simulink model "ClimaTiMgr"  (R2022b)
settings: Solver=FixedStepDiscrete  StartTime=0.0  StopTime=10.0  FixedStep=0.1  RelTol=1e-3
settings Model: Creator=blue.ge  LastModifiedBy=blue.ge
settings DataIOCC: SaveFormat=StructureWithTime
settings OptimizationCC: DefaultParameterBehavior=Inlined  EnhancedBackFolding=on  PassReuseOutputArgsAs=Individual arguments  ZeroExternalMemoryAtStartup=off  ZeroInternalMemoryAtStartup=off  OptimizeBlockOrder=speed  DifferentSizesBufferReuse=on  OptimizationCustomize=off  EfficientTunableParamExpr=on
settings DebuggingCC: ParameterTunabilityLossMsg=error
settings ModelReferenceCC: ModelReferenceNumInstancesAllowed=Single
settings SFSimCC: MATLABDynamicMemAlloc=off
settings RTWCC: Description=AUTOSAR  SystemTargetFile=autosar.tlc  GenCodeOnly=on  TemplateMakefile=ert_default_tmf
settings CodeAppCC: ForceParamTrailComments=on  IgnoreCustomStorageClasses=off  OperatorAnnotations=on  SimulinkDataObjDesc=on  SFDataObjDesc=on  InsertBlockDesc=on
settings STFCustomTargetCC: TargetLangStandard=C99 (ISO)  MultiwordLength=256  ParMdlRefBuildCompliant=on  CompOptLevelCompliant=on  IncludeMdlTerminateFcn=off  CombineOutputUpdateFcns=on  SuppressErrorStatus=on  ERTCustomFileBanners=on  SupportNonFinite=off  SupportComplex=off  SupportContinuousTime=off  SupportNonInlinedSFcns=off  RemoveResetFunc=on  MATLABClassNameForMDSCustomization=Simulink.SoftwareTarget.ERTCustomization  AutosarCompliant=on  UseToolchainInfoCompliant=on  LookupTableObjectStructAxisOrder=2,1,3,4,...  LUTObjectStructOrderEvenSpacing=Size,Table,Breakpoints  SystemTargetFile=autosar.tlc
settings ERTTargetCC: TargetLangStandard=C99 (ISO)  ConcurrentExecutionCompliant=off  IncludeMdlTerminateFcn=off  SuppressErrorStatus=on  SupportNonFinite=off  SupportComplex=off  ModelStepFunctionPrototypeControlCompliant=off  CPPClassGenCompliant=off  AutosarCompliant=on  LookupTableObjectStructAxisOrder=2,1,3,4,...  LUTObjectStructOrderEvenSpacing=Size,Table,Breakpoints  InlinedParameterPlacement=NonHierarchical  ERTCustomFileTemplate=example_file_process.tlc

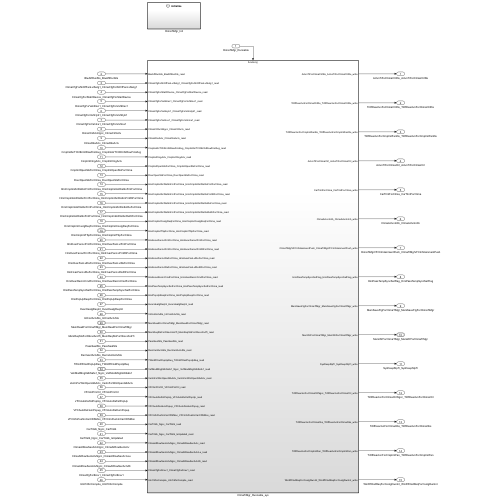
[thumbnail: Simulink's stored preview of the top-level diagram]
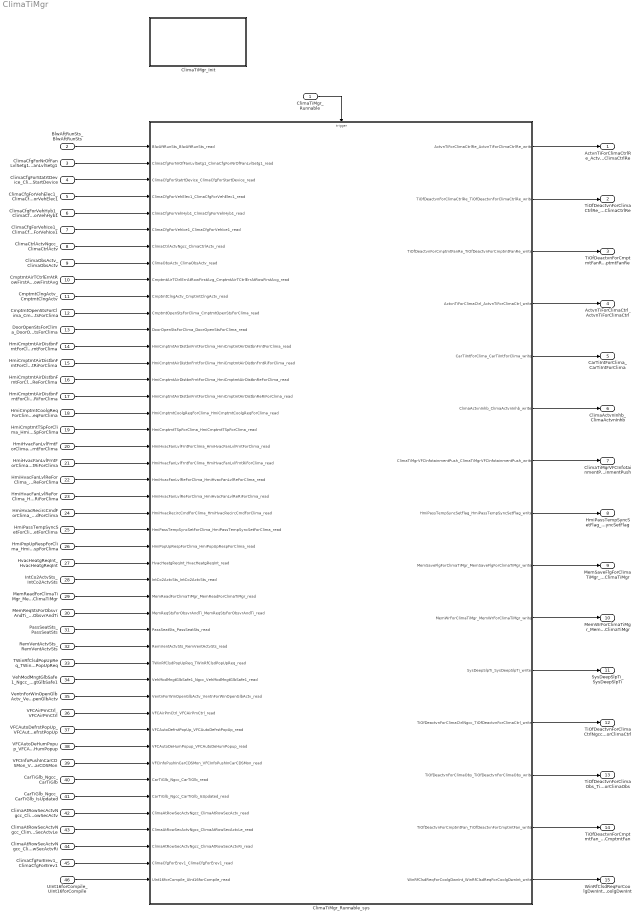
== system ClimaTiMgr ==
  ClimaTiMgr_Runnable : Inport  SampleTime=0.1  OutputFunctionCall=on
  BlwAftRunSts_BlwAftRunSts : Inport  Port=2  OutDataTypeStr=Boolean  PortDimensions=1
  ClimaCfgForNrOfFanLvlSetg1_ClimaCfgForNrOfFanLvlSetg1 : Inport  Port=3  OutDataTypeStr=Enum: ClimaCfgForNrOfFanLvlSetg1  PortDimensions=1
  ClimaCfgForStatrtDevice_ClimaCfgForStartDevice : Inport  Port=4  OutDataTypeStr=Enum: StartDevice  PortDimensions=1
  ClimaCfgForVehElec1_ClimaCfgForVehElec1 : Inport  Port=5  OutDataTypeStr=Boolean  PortDimensions=1
  ClimaCfgForVehHyb1_ClimaCfgForVehHyb1 : Inport  Port=6  OutDataTypeStr=Boolean  PortDimensions=1
  ClimaCfgForVehIce1_ClimaCfgForVehIce1 : Inport  Port=7  OutDataTypeStr=Boolean  PortDimensions=1
  ClimaCtrlActvNgcc_ClimaCtrlActv : Inport  Port=8  OutDataTypeStr=Boolean  PortDimensions=1
  ClimaObsActv_ClimaObsActv : Inport  Port=9  OutDataTypeStr=Boolean  PortDimensions=1
  CmptmtAirTCtrlErrAtRowFirstAvg_CmptmtAirTCtrlErrAtRowFirstAvg : Inport  Port=10  OutDataTypeStr=T30  PortDimensions=1
  CmptmtClngActv_CmptmtClngActv : Inport  Port=11  OutDataTypeStr=Enum: CmptmtClngActv  PortDimensions=1
  CmptmtOpenStsForClima_CmptmtOpenStsForClima : Inport  Port=12  OutDataTypeStr=Boolean  PortDimensions=1
  DoorOpenStsForClima_DoorOpenStsForClima : Inport  Port=13  OutDataTypeStr=Boolean  PortDimensions=1
  HmiCmptmtAirDistbnFrntForClima_HmiCmptmtAirDistbnFrntForClima : Inport  Port=14  OutDataTypeStr=Enum: HmiCmptmtAirDistbnFrnt  PortDimensions=1
  HmiCmptmtAirDistbnFrntForClima_HmiCmptmtAirDistbnFrntRiForClima : Inport  Port=15  OutDataTypeStr=Enum: HmiCmptmtAirDistbnFrnt  PortDimensions=1
  HmiCmptmtAirDistbnFrntForClima_HmiCmptmtAirDistbnReForClima : Inport  Port=16  OutDataTypeStr=Enum: HmiCmptmtAirDistbnFrnt  PortDimensions=1
  HmiCmptmtAirDistbnFrntForClima_HmiCmptmtAirDistbnReRiForClima : Inport  Port=17  OutDataTypeStr=Enum: HmiCmptmtAirDistbnFrnt  PortDimensions=1
  HmiCmptmtCoolgReqForClima_HmiCmptmtCoolgReqForClima : Inport  Port=18  OutDataTypeStr=Enum: HmiCmptmtCoolgReq  PortDimensions=1
  HmiCmptmtTSpForClima_HmiCmptmtTSpForClima : Inport  Port=19  OutDataTypeStr=Bus: HmiCmptmtTSp  PortDimensions=1  BusOutputAsStruct=on
  HmiHvacFanLvlFrntForClima_HmiHvacFanLvlFrntForClima : Inport  Port=20  OutDataTypeStr=Enum: HmiHvacFanLvl  PortDimensions=1
  HmiHvacFanLvlFrntForClima_HmiHvacFanLvlFrntRiForClima : Inport  Port=21  OutDataTypeStr=Enum: HmiHvacFanLvl  PortDimensions=1
  HmiHvacFanLvlReForClima_HmiHvacFanLvlReForClima : Inport  Port=22  OutDataTypeStr=Enum: HmiHvacFanLvl  PortDimensions=1
  HmiHvacFanLvlReForClima_HmiHvacFanLvlReRiForClima : Inport  Port=23  OutDataTypeStr=Enum: HmiHvacFanLvl  PortDimensions=1
  HmiHvacRecircCmdForClima_HmiHvacRecircCmdForClima : Inport  Port=24  OutDataTypeStr=Enum: HmiHvacRecircCmd  PortDimensions=1
  HmiPassTempSyncSetForClima_HmiPassTempSyncSetForClima : Inport  Port=25  OutDataTypeStr=Enum: OnOff1  PortDimensions=1
  HmiPopUpRespForClima_HmiPopUpRespForClima : Inport  Port=26  OutDataTypeStr=Enum: BookChargeSetResponse  PortDimensions=1
  HvacHeatgReqInt_HvacHeatgReqInt : Inport  Port=27  OutDataTypeStr=Enum: OnOff1  PortDimensions=1
  IntCo2ActvSts_IntCo2ActvSts : Inport  Port=28  OutDataTypeStr=Enum: IntCo2Sts  PortDimensions=1
  MemReadForClimaTiMgr_MemReadForClimaTiMgr : Inport  Port=29  OutDataTypeStr=Bus: MemRecForClimaTiMgr  PortDimensions=1  BusOutputAsStruct=on
  MemReqStsForObsvrAndTi_MemReqStsForObsvrAndTi : Inport  Port=30  OutDataTypeStr=Enum: MemReqSts  PortDimensions=1
  PassSeatSts_PassSeatSts : Inport  Port=31  OutDataTypeStr=Enum: PassSeatSts  PortDimensions=1
  RemVentActvSts_RemVentActvSts : Inport  Port=32  OutDataTypeStr=Enum: OnOff1  PortDimensions=1
  TWinRfClsdPopUpReq_TWinRfClsdPopUpReq : Inport  Port=33  OutDataTypeStr=Enum: OnOff1  PortDimensions=1
  VehModMngtGlbSafe1_Ngcc_VehModMngtGlbSafe1 : Inport  Port=34  OutDataTypeStr=Bus: VehModMngtGlbSafe1  PortDimensions=1  BusOutputAsStruct=on
  VentnForWinOpenGlbActv_VentnForWinOpenGlbActv : Inport  Port=35  OutDataTypeStr=Boolean  PortDimensions=1
  VFCAirPmCtrl_VFCAirPmCtrl : Inport  Port=36  OutDataTypeStr=Enum: OnOff1  PortDimensions=1
  VFCAutoDefrstPopUp_VFCAutoDefrstPopUp : Inport  Port=37  OutDataTypeStr=Boolean  PortDimensions=1
  VFCAutoDeHumPopup_VFCAutoDeHumPopup : Inport  Port=38  OutDataTypeStr=Boolean  PortDimensions=1
  VFCInfoPushInCarCDSMon_VFCInfoPushInCarCDSMon : Inport  Port=39  OutDataTypeStr=Boolean  PortDimensions=1
  CarTiGlb_Ngcc_CarTiGlb : Inport  Port=40  OutDataTypeStr=CarTiGlb  PortDimensions=1
  CarTiGlb_Ngcc_CarTiGlb_IsUpdated : Inport  Port=41  OutDataTypeStr=boolean  PortDimensions=1
  ClimaAtRowSecActvNgcc_ClimaAtRowSecActv : Inport  Port=42  OutDataTypeStr=Boolean  PortDimensions=1
  ClimaAtRowSecActvNgcc_ClimaAtRowSecActvLe : Inport  Port=43  OutDataTypeStr=Boolean  PortDimensions=1
  ClimaAtRowSecActvNgcc_ClimaAtRowSecActvRi : Inport  Port=44  OutDataTypeStr=Boolean  PortDimensions=1
  ClimaCfgForErev1_ClimaCfgForErev1 : Inport  Port=45  OutDataTypeStr=Boolean  PortDimensions=1
  UInt16forCompile_UInt16forCompile : Inport  Port=46  OutDataTypeStr=UInt16  PortDimensions=1
  ClimaTiMgr_Init : Subsystem
  ClimaTiMgr_Runnable_sys : Subsystem
  ActvnTiForClimaCtrlRe_ActvnTiForClimaCtrlRe : Outport  OutDataTypeStr=Ti33  PortDimensions=1
  TiOfDeactvnForClimaCtrlRe_TiOfDeactvnForClimaCtrlRe : Outport  Port=2  OutDataTypeStr=Ti33  PortDimensions=1
  TiOfDeactvnForCmptmtFanRe_TiOfDeactvnForCmptmtFanRe : Outport  Port=3  OutDataTypeStr=Ti33  PortDimensions=1
  ActvnTiForClimaCtrl_ActvnTiForClimaCtrl : Outport  Port=4  OutDataTypeStr=Ti33  PortDimensions=1
  CarTiIntForClima_CarTiIntForClima : Outport  Port=5  OutDataTypeStr=CarTiGlb  PortDimensions=1
  ClimaActvnInhb_ClimaActvnInhb : Outport  Port=6  OutDataTypeStr=Enum: OnOff1  PortDimensions=1
  ClimaTiMgrVFCInfotainmentPush_ClimaTiMgrVFCInfotainmentPush : Outport  Port=7  OutDataTypeStr=Enum: OnOff1  PortDimensions=1
  HmiPassTempSyncSetFlag_HmiPassTempSyncSetFlag : Outport  Port=8  OutDataTypeStr=Boolean  PortDimensions=1
  MemSaveFlgForClimaTiMgr_MemSaveFlgForClimaTiMgr : Outport  Port=9  OutDataTypeStr=Boolean  PortDimensions=1
  MemWrForClimaTiMgr_MemWrForClimaTiMgr : Outport  Port=10  OutDataTypeStr=Bus: MemRecForClimaTiMgr  PortDimensions=1  BusOutputAsStruct=on
  SysDeepSlpTi_SysDeepSlpTi : Outport  Port=11  OutDataTypeStr=CarTiGlb  PortDimensions=1
  TiOfDeactvnForClimaCtrlNgcc_TiOfDeactvnForClimaCtrl : Outport  Port=12  OutDataTypeStr=Ti33  PortDimensions=1
  TiOfDeactvnForClimaObs_TiOfDeactvnForClimaObs : Outport  Port=13  OutDataTypeStr=Ti33  PortDimensions=1
  TiOfDeactvnForCmptmtFan_TiOfDeactvnForCmptmtFan : Outport  Port=14  OutDataTypeStr=Ti33  PortDimensions=1
  WinRfClsdReqForCoolgDwnInt_WinRfClsdReqForCoolgDwnInt : Outport  Port=15  OutDataTypeStr=Enum: Warn2  PortDimensions=1
  wires:
  BlwAftRunSts_BlwAftRunSts/1 -> ClimaTiMgr_Runnable_sys/1(BlwAftRunSts_BlwAftRunSts_read)
  ClimaCfgForNrOfFanLvlSetg1_ClimaCfgForNrOfFanLvlSetg1/1 -> ClimaTiMgr_Runnable_sys/2(ClimaCfgForNrOfFanLvlSetg1_ClimaCfgForNrOfFanLvlSetg1_read)
  ClimaCfgForStatrtDevice_ClimaCfgForStartDevice/1 -> ClimaTiMgr_Runnable_sys/3(ClimaCfgForStatrtDevice_ClimaCfgForStartDevice_read)
  ClimaCfgForVehElec1_ClimaCfgForVehElec1/1 -> ClimaTiMgr_Runnable_sys/4(ClimaCfgForVehElec1_ClimaCfgForVehElec1_read)
  ClimaCfgForVehHyb1_ClimaCfgForVehHyb1/1 -> ClimaTiMgr_Runnable_sys/5(ClimaCfgForVehHyb1_ClimaCfgForVehHyb1_read)
  ClimaCfgForVehIce1_ClimaCfgForVehIce1/1 -> ClimaTiMgr_Runnable_sys/6(ClimaCfgForVehIce1_ClimaCfgForVehIce1_read)
  ClimaCtrlActvNgcc_ClimaCtrlActv/1 -> ClimaTiMgr_Runnable_sys/7(ClimaCtrlActvNgcc_ClimaCtrlActv_read)
  ClimaObsActv_ClimaObsActv/1 -> ClimaTiMgr_Runnable_sys/8(ClimaObsActv_ClimaObsActv_read)
  CmptmtAirTCtrlErrAtRowFirstAvg_CmptmtAirTCtrlErrAtRowFirstAvg/1 -> ClimaTiMgr_Runnable_sys/9(CmptmtAirTCtrlErrAtRowFirstAvg_CmptmtAirTCtrlErrAtRowFirstAvg_read)
  CmptmtClngActv_CmptmtClngActv/1 -> ClimaTiMgr_Runnable_sys/10(CmptmtClngActv_CmptmtClngActv_read)
  CmptmtOpenStsForClima_CmptmtOpenStsForClima/1 -> ClimaTiMgr_Runnable_sys/11(CmptmtOpenStsForClima_CmptmtOpenStsForClima_read)
  DoorOpenStsForClima_DoorOpenStsForClima/1 -> ClimaTiMgr_Runnable_sys/12(DoorOpenStsForClima_DoorOpenStsForClima_read)
  HmiCmptmtAirDistbnFrntForClima_HmiCmptmtAirDistbnFrntForClima/1 -> ClimaTiMgr_Runnable_sys/13(HmiCmptmtAirDistbnFrntForClima_HmiCmptmtAirDistbnFrntForClima_read)
  HmiCmptmtAirDistbnFrntForClima_HmiCmptmtAirDistbnFrntRiForClima/1 -> ClimaTiMgr_Runnable_sys/14(HmiCmptmtAirDistbnFrntForClima_HmiCmptmtAirDistbnFrntRiForClima_read)
  HmiCmptmtAirDistbnFrntForClima_HmiCmptmtAirDistbnReForClima/1 -> ClimaTiMgr_Runnable_sys/15(HmiCmptmtAirDistbnFrntForClima_HmiCmptmtAirDistbnReForClima_read)
  HmiCmptmtAirDistbnFrntForClima_HmiCmptmtAirDistbnReRiForClima/1 -> ClimaTiMgr_Runnable_sys/16(HmiCmptmtAirDistbnFrntForClima_HmiCmptmtAirDistbnReRiForClima_read)
  HmiCmptmtCoolgReqForClima_HmiCmptmtCoolgReqForClima/1 -> ClimaTiMgr_Runnable_sys/17(HmiCmptmtCoolgReqForClima_HmiCmptmtCoolgReqForClima_read)
  HmiCmptmtTSpForClima_HmiCmptmtTSpForClima/1 -> ClimaTiMgr_Runnable_sys/18(HmiCmptmtTSpForClima_HmiCmptmtTSpForClima_read)
  HmiHvacFanLvlFrntForClima_HmiHvacFanLvlFrntForClima/1 -> ClimaTiMgr_Runnable_sys/19(HmiHvacFanLvlFrntForClima_HmiHvacFanLvlFrntForClima_read)
  HmiHvacFanLvlFrntForClima_HmiHvacFanLvlFrntRiForClima/1 -> ClimaTiMgr_Runnable_sys/20(HmiHvacFanLvlFrntForClima_HmiHvacFanLvlFrntRiForClima_read)
  HmiHvacFanLvlReForClima_HmiHvacFanLvlReForClima/1 -> ClimaTiMgr_Runnable_sys/21(HmiHvacFanLvlReForClima_HmiHvacFanLvlReForClima_read)
  HmiHvacFanLvlReForClima_HmiHvacFanLvlReRiForClima/1 -> ClimaTiMgr_Runnable_sys/22(HmiHvacFanLvlReForClima_HmiHvacFanLvlReRiForClima_read)
  HmiHvacRecircCmdForClima_HmiHvacRecircCmdForClima/1 -> ClimaTiMgr_Runnable_sys/23(HmiHvacRecircCmdForClima_HmiHvacRecircCmdForClima_read)
  HmiPassTempSyncSetForClima_HmiPassTempSyncSetForClima/1 -> ClimaTiMgr_Runnable_sys/24(HmiPassTempSyncSetForClima_HmiPassTempSyncSetForClima_read)
  HmiPopUpRespForClima_HmiPopUpRespForClima/1 -> ClimaTiMgr_Runnable_sys/25(HmiPopUpRespForClima_HmiPopUpRespForClima_read)
  HvacHeatgReqInt_HvacHeatgReqInt/1 -> ClimaTiMgr_Runnable_sys/26(HvacHeatgReqInt_HvacHeatgReqInt_read)
  IntCo2ActvSts_IntCo2ActvSts/1 -> ClimaTiMgr_Runnable_sys/27(IntCo2ActvSts_IntCo2ActvSts_read)
  MemReadForClimaTiMgr_MemReadForClimaTiMgr/1 -> ClimaTiMgr_Runnable_sys/28(MemReadForClimaTiMgr_MemReadForClimaTiMgr_read)
  MemReqStsForObsvrAndTi_MemReqStsForObsvrAndTi/1 -> ClimaTiMgr_Runnable_sys/29(MemReqStsForObsvrAndTi_MemReqStsForObsvrAndTi_read)
  PassSeatSts_PassSeatSts/1 -> ClimaTiMgr_Runnable_sys/30(PassSeatSts_PassSeatSts_read)
  RemVentActvSts_RemVentActvSts/1 -> ClimaTiMgr_Runnable_sys/31(RemVentActvSts_RemVentActvSts_read)
  TWinRfClsdPopUpReq_TWinRfClsdPopUpReq/1 -> ClimaTiMgr_Runnable_sys/32(TWinRfClsdPopUpReq_TWinRfClsdPopUpReq_read)
  VehModMngtGlbSafe1_Ngcc_VehModMngtGlbSafe1/1 -> ClimaTiMgr_Runnable_sys/33(VehModMngtGlbSafe1_Ngcc_VehModMngtGlbSafe1_read)
  VentnForWinOpenGlbActv_VentnForWinOpenGlbActv/1 -> ClimaTiMgr_Runnable_sys/34(VentnForWinOpenGlbActv_VentnForWinOpenGlbActv_read)
  VFCAirPmCtrl_VFCAirPmCtrl/1 -> ClimaTiMgr_Runnable_sys/35(VFCAirPmCtrl_VFCAirPmCtrl_read)
  VFCAutoDefrstPopUp_VFCAutoDefrstPopUp/1 -> ClimaTiMgr_Runnable_sys/36(VFCAutoDefrstPopUp_VFCAutoDefrstPopUp_read)
  VFCAutoDeHumPopup_VFCAutoDeHumPopup/1 -> ClimaTiMgr_Runnable_sys/37(VFCAutoDeHumPopup_VFCAutoDeHumPopup_read)
  VFCInfoPushInCarCDSMon_VFCInfoPushInCarCDSMon/1 -> ClimaTiMgr_Runnable_sys/38(VFCInfoPushInCarCDSMon_VFCInfoPushInCarCDSMon_read)
  CarTiGlb_Ngcc_CarTiGlb/1 -> ClimaTiMgr_Runnable_sys/39(CarTiGlb_Ngcc_CarTiGlb_read)
  CarTiGlb_Ngcc_CarTiGlb_IsUpdated/1 -> ClimaTiMgr_Runnable_sys/40(CarTiGlb_Ngcc_CarTiGlb_IsUpdated_read)
  ClimaAtRowSecActvNgcc_ClimaAtRowSecActv/1 -> ClimaTiMgr_Runnable_sys/41(ClimaAtRowSecActvNgcc_ClimaAtRowSecActv_read)
  ClimaAtRowSecActvNgcc_ClimaAtRowSecActvLe/1 -> ClimaTiMgr_Runnable_sys/42(ClimaAtRowSecActvNgcc_ClimaAtRowSecActvLe_read)
  ClimaAtRowSecActvNgcc_ClimaAtRowSecActvRi/1 -> ClimaTiMgr_Runnable_sys/43(ClimaAtRowSecActvNgcc_ClimaAtRowSecActvRi_read)
  ClimaCfgForErev1_ClimaCfgForErev1/1 -> ClimaTiMgr_Runnable_sys/44(ClimaCfgForErev1_ClimaCfgForErev1_read)
  UInt16forCompile_UInt16forCompile/1 -> ClimaTiMgr_Runnable_sys/45(UInt16forCompile_UInt16forCompile_read)
  ClimaTiMgr_Runnable_sys/1(ActvnTiForClimaCtrlRe_ActvnTiForClimaCtrlRe_write) -> ActvnTiForClimaCtrlRe_ActvnTiForClimaCtrlRe/1
  ClimaTiMgr_Runnable_sys/2(TiOfDeactvnForClimaCtrlRe_TiOfDeactvnForClimaCtrlRe_write) -> TiOfDeactvnForClimaCtrlRe_TiOfDeactvnForClimaCtrlRe/1
  ClimaTiMgr_Runnable_sys/3(TiOfDeactvnForCmptmtFanRe_TiOfDeactvnForCmptmtFanRe_write) -> TiOfDeactvnForCmptmtFanRe_TiOfDeactvnForCmptmtFanRe/1
  ClimaTiMgr_Runnable_sys/4(ActvnTiForClimaCtrl_ActvnTiForClimaCtrl_write) -> ActvnTiForClimaCtrl_ActvnTiForClimaCtrl/1
  ClimaTiMgr_Runnable_sys/5(CarTiIntForClima_CarTiIntForClima_write) -> CarTiIntForClima_CarTiIntForClima/1
  ClimaTiMgr_Runnable_sys/6(ClimaActvnInhb_ClimaActvnInhb_write) -> ClimaActvnInhb_ClimaActvnInhb/1
  ClimaTiMgr_Runnable_sys/7(ClimaTiMgrVFCInfotainmentPush_ClimaTiMgrVFCInfotainmentPush_write) -> ClimaTiMgrVFCInfotainmentPush_ClimaTiMgrVFCInfotainmentPush/1
  ClimaTiMgr_Runnable_sys/8(HmiPassTempSyncSetFlag_HmiPassTempSyncSetFlag_write) -> HmiPassTempSyncSetFlag_HmiPassTempSyncSetFlag/1
  ClimaTiMgr_Runnable_sys/9(MemSaveFlgForClimaTiMgr_MemSaveFlgForClimaTiMgr_write) -> MemSaveFlgForClimaTiMgr_MemSaveFlgForClimaTiMgr/1
  ClimaTiMgr_Runnable_sys/10(MemWrForClimaTiMgr_MemWrForClimaTiMgr_write) -> MemWrForClimaTiMgr_MemWrForClimaTiMgr/1
  ClimaTiMgr_Runnable_sys/11(SysDeepSlpTi_SysDeepSlpTi_write) -> SysDeepSlpTi_SysDeepSlpTi/1
  ClimaTiMgr_Runnable_sys/12(TiOfDeactvnForClimaCtrlNgcc_TiOfDeactvnForClimaCtrl_write) -> TiOfDeactvnForClimaCtrlNgcc_TiOfDeactvnForClimaCtrl/1
  ClimaTiMgr_Runnable_sys/13(TiOfDeactvnForClimaObs_TiOfDeactvnForClimaObs_write) -> TiOfDeactvnForClimaObs_TiOfDeactvnForClimaObs/1
  ClimaTiMgr_Runnable_sys/14(TiOfDeactvnForCmptmtFan_TiOfDeactvnForCmptmtFan_write) -> TiOfDeactvnForCmptmtFan_TiOfDeactvnForCmptmtFan/1
  ClimaTiMgr_Runnable_sys/15(WinRfClsdReqForCoolgDwnInt_WinRfClsdReqForCoolgDwnInt_write) -> WinRfClsdReqForCoolgDwnInt_WinRfClsdReqForCoolgDwnInt/1
  ClimaTiMgr_Runnable/1 -> ClimaTiMgr_Runnable_sys/Trigger

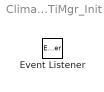
== system ClimaTiMgr/ClimaTiMgr_Init ==
  Event Listener : EventListener  EventName=reset

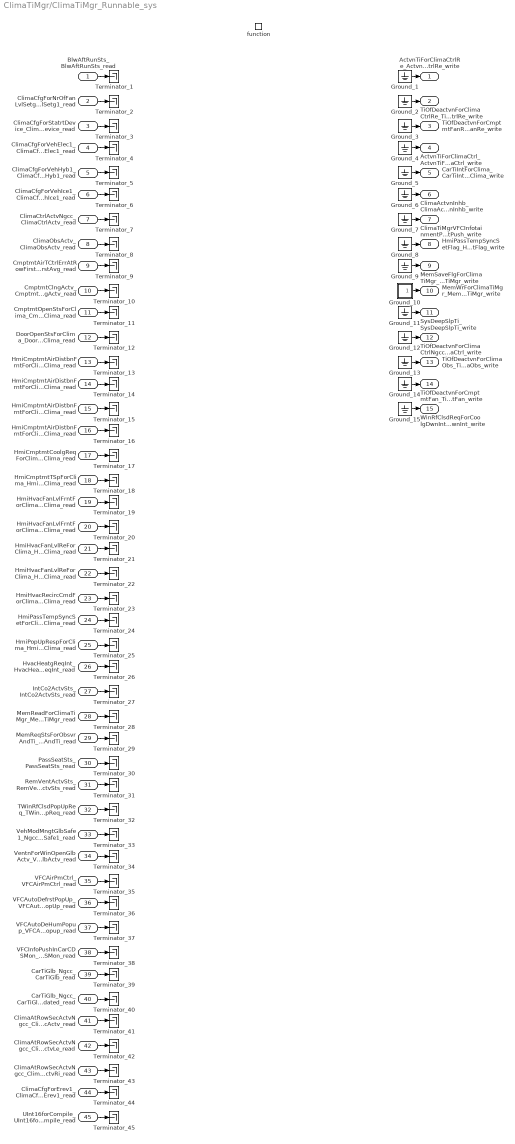
== system ClimaTiMgr/ClimaTiMgr_Runnable_sys ==
  BlwAftRunSts_BlwAftRunSts_read : Inport
  ClimaCfgForNrOfFanLvlSetg1_ClimaCfgForNrOfFanLvlSetg1_read : Inport  Port=2
  ClimaCfgForStatrtDevice_ClimaCfgForStartDevice_read : Inport  Port=3
  ClimaCfgForVehElec1_ClimaCfgForVehElec1_read : Inport  Port=4
  ClimaCfgForVehHyb1_ClimaCfgForVehHyb1_read : Inport  Port=5
  ClimaCfgForVehIce1_ClimaCfgForVehIce1_read : Inport  Port=6
  ClimaCtrlActvNgcc_ClimaCtrlActv_read : Inport  Port=7
  ClimaObsActv_ClimaObsActv_read : Inport  Port=8
  CmptmtAirTCtrlErrAtRowFirstAvg_CmptmtAirTCtrlErrAtRowFirstAvg_read : Inport  Port=9
  CmptmtClngActv_CmptmtClngActv_read : Inport  Port=10
  CmptmtOpenStsForClima_CmptmtOpenStsForClima_read : Inport  Port=11
  DoorOpenStsForClima_DoorOpenStsForClima_read : Inport  Port=12
  HmiCmptmtAirDistbnFrntForClima_HmiCmptmtAirDistbnFrntForClima_read : Inport  Port=13
  HmiCmptmtAirDistbnFrntForClima_HmiCmptmtAirDistbnFrntRiForClima_read : Inport  Port=14
  HmiCmptmtAirDistbnFrntForClima_HmiCmptmtAirDistbnReForClima_read : Inport  Port=15
  HmiCmptmtAirDistbnFrntForClima_HmiCmptmtAirDistbnReRiForClima_read : Inport  Port=16
  HmiCmptmtCoolgReqForClima_HmiCmptmtCoolgReqForClima_read : Inport  Port=17
  HmiCmptmtTSpForClima_HmiCmptmtTSpForClima_read : Inport  Port=18
  HmiHvacFanLvlFrntForClima_HmiHvacFanLvlFrntForClima_read : Inport  Port=19
  HmiHvacFanLvlFrntForClima_HmiHvacFanLvlFrntRiForClima_read : Inport  Port=20
  HmiHvacFanLvlReForClima_HmiHvacFanLvlReForClima_read : Inport  Port=21
  HmiHvacFanLvlReForClima_HmiHvacFanLvlReRiForClima_read : Inport  Port=22
  HmiHvacRecircCmdForClima_HmiHvacRecircCmdForClima_read : Inport  Port=23
  HmiPassTempSyncSetForClima_HmiPassTempSyncSetForClima_read : Inport  Port=24
  HmiPopUpRespForClima_HmiPopUpRespForClima_read : Inport  Port=25
  HvacHeatgReqInt_HvacHeatgReqInt_read : Inport  Port=26
  IntCo2ActvSts_IntCo2ActvSts_read : Inport  Port=27
  MemReadForClimaTiMgr_MemReadForClimaTiMgr_read : Inport  Port=28
  MemReqStsForObsvrAndTi_MemReqStsForObsvrAndTi_read : Inport  Port=29
  PassSeatSts_PassSeatSts_read : Inport  Port=30
  RemVentActvSts_RemVentActvSts_read : Inport  Port=31
  TWinRfClsdPopUpReq_TWinRfClsdPopUpReq_read : Inport  Port=32
  VehModMngtGlbSafe1_Ngcc_VehModMngtGlbSafe1_read : Inport  Port=33
  VentnForWinOpenGlbActv_VentnForWinOpenGlbActv_read : Inport  Port=34
  VFCAirPmCtrl_VFCAirPmCtrl_read : Inport  Port=35
  VFCAutoDefrstPopUp_VFCAutoDefrstPopUp_read : Inport  Port=36
  VFCAutoDeHumPopup_VFCAutoDeHumPopup_read : Inport  Port=37
  VFCInfoPushInCarCDSMon_VFCInfoPushInCarCDSMon_read : Inport  Port=38
  CarTiGlb_Ngcc_CarTiGlb_read : Inport  Port=39
  CarTiGlb_Ngcc_CarTiGlb_IsUpdated_read : Inport  Port=40
  ClimaAtRowSecActvNgcc_ClimaAtRowSecActv_read : Inport  Port=41
  ClimaAtRowSecActvNgcc_ClimaAtRowSecActvLe_read : Inport  Port=42
  ClimaAtRowSecActvNgcc_ClimaAtRowSecActvRi_read : Inport  Port=43
  ClimaCfgForErev1_ClimaCfgForErev1_read : Inport  Port=44
  UInt16forCompile_UInt16forCompile_read : Inport  Port=45
  function : TriggerPort  TriggerType=function-call  VariantControl=(inherit)  SampleTimeType=periodic  SampleTime=-1
  Ground_{1..9,11..15} (14 alike) : Ground
  Ground_10 : Subsystem
  Terminator_{1..45} (45 alike) : Terminator
  ActvnTiForClimaCtrlRe_ActvnTiForClimaCtrlRe_write : Outport
      settings: EnsureOutportIsVirtual=on
  TiOfDeactvnForClimaCtrlRe_TiOfDeactvnForClimaCtrlRe_write : Outport  Port=2
      settings: EnsureOutportIsVirtual=on
  TiOfDeactvnForCmptmtFanRe_TiOfDeactvnForCmptmtFanRe_write : Outport  Port=3
      settings: EnsureOutportIsVirtual=on
  ActvnTiForClimaCtrl_ActvnTiForClimaCtrl_write : Outport  Port=4
      settings: EnsureOutportIsVirtual=on
  CarTiIntForClima_CarTiIntForClima_write : Outport  Port=5
      settings: EnsureOutportIsVirtual=on
  ClimaActvnInhb_ClimaActvnInhb_write : Outport  Port=6
      settings: EnsureOutportIsVirtual=on
  ClimaTiMgrVFCInfotainmentPush_ClimaTiMgrVFCInfotainmentPush_write : Outport  Port=7
      settings: EnsureOutportIsVirtual=on
  HmiPassTempSyncSetFlag_HmiPassTempSyncSetFlag_write : Outport  Port=8
      settings: EnsureOutportIsVirtual=on
  MemSaveFlgForClimaTiMgr_MemSaveFlgForClimaTiMgr_write : Outport  Port=9
      settings: EnsureOutportIsVirtual=on
  MemWrForClimaTiMgr_MemWrForClimaTiMgr_write : Outport  Port=10
      settings: EnsureOutportIsVirtual=on
  SysDeepSlpTi_SysDeepSlpTi_write : Outport  Port=11
      settings: EnsureOutportIsVirtual=on
  TiOfDeactvnForClimaCtrlNgcc_TiOfDeactvnForClimaCtrl_write : Outport  Port=12
      settings: EnsureOutportIsVirtual=on
  TiOfDeactvnForClimaObs_TiOfDeactvnForClimaObs_write : Outport  Port=13
      settings: EnsureOutportIsVirtual=on
  TiOfDeactvnForCmptmtFan_TiOfDeactvnForCmptmtFan_write : Outport  Port=14
      settings: EnsureOutportIsVirtual=on
  WinRfClsdReqForCoolgDwnInt_WinRfClsdReqForCoolgDwnInt_write : Outport  Port=15
      settings: EnsureOutportIsVirtual=on
  wires:
  BlwAftRunSts_BlwAftRunSts_read/1 -> Terminator_1/1
  ClimaCfgForNrOfFanLvlSetg1_ClimaCfgForNrOfFanLvlSetg1_read/1 -> Terminator_2/1
  ClimaCfgForStatrtDevice_ClimaCfgForStartDevice_read/1 -> Terminator_3/1
  ClimaCfgForVehElec1_ClimaCfgForVehElec1_read/1 -> Terminator_4/1
  ClimaCfgForVehHyb1_ClimaCfgForVehHyb1_read/1 -> Terminator_5/1
  ClimaCfgForVehIce1_ClimaCfgForVehIce1_read/1 -> Terminator_6/1
  ClimaCtrlActvNgcc_ClimaCtrlActv_read/1 -> Terminator_7/1
  ClimaObsActv_ClimaObsActv_read/1 -> Terminator_8/1
  CmptmtAirTCtrlErrAtRowFirstAvg_CmptmtAirTCtrlErrAtRowFirstAvg_read/1 -> Terminator_9/1
  CmptmtClngActv_CmptmtClngActv_read/1 -> Terminator_10/1
  CmptmtOpenStsForClima_CmptmtOpenStsForClima_read/1 -> Terminator_11/1
  DoorOpenStsForClima_DoorOpenStsForClima_read/1 -> Terminator_12/1
  HmiCmptmtAirDistbnFrntForClima_HmiCmptmtAirDistbnFrntForClima_read/1 -> Terminator_13/1
  HmiCmptmtAirDistbnFrntForClima_HmiCmptmtAirDistbnFrntRiForClima_read/1 -> Terminator_14/1
  HmiCmptmtAirDistbnFrntForClima_HmiCmptmtAirDistbnReForClima_read/1 -> Terminator_15/1
  HmiCmptmtAirDistbnFrntForClima_HmiCmptmtAirDistbnReRiForClima_read/1 -> Terminator_16/1
  HmiCmptmtCoolgReqForClima_HmiCmptmtCoolgReqForClima_read/1 -> Terminator_17/1
  HmiCmptmtTSpForClima_HmiCmptmtTSpForClima_read/1 -> Terminator_18/1
  HmiHvacFanLvlFrntForClima_HmiHvacFanLvlFrntForClima_read/1 -> Terminator_19/1
  HmiHvacFanLvlFrntForClima_HmiHvacFanLvlFrntRiForClima_read/1 -> Terminator_20/1
  HmiHvacFanLvlReForClima_HmiHvacFanLvlReForClima_read/1 -> Terminator_21/1
  HmiHvacFanLvlReForClima_HmiHvacFanLvlReRiForClima_read/1 -> Terminator_22/1
  HmiHvacRecircCmdForClima_HmiHvacRecircCmdForClima_read/1 -> Terminator_23/1
  HmiPassTempSyncSetForClima_HmiPassTempSyncSetForClima_read/1 -> Terminator_24/1
  HmiPopUpRespForClima_HmiPopUpRespForClima_read/1 -> Terminator_25/1
  HvacHeatgReqInt_HvacHeatgReqInt_read/1 -> Terminator_26/1
  IntCo2ActvSts_IntCo2ActvSts_read/1 -> Terminator_27/1
  MemReadForClimaTiMgr_MemReadForClimaTiMgr_read/1 -> Terminator_28/1
  MemReqStsForObsvrAndTi_MemReqStsForObsvrAndTi_read/1 -> Terminator_29/1
  PassSeatSts_PassSeatSts_read/1 -> Terminator_30/1
  RemVentActvSts_RemVentActvSts_read/1 -> Terminator_31/1
  TWinRfClsdPopUpReq_TWinRfClsdPopUpReq_read/1 -> Terminator_32/1
  VehModMngtGlbSafe1_Ngcc_VehModMngtGlbSafe1_read/1 -> Terminator_33/1
  VentnForWinOpenGlbActv_VentnForWinOpenGlbActv_read/1 -> Terminator_34/1
  VFCAirPmCtrl_VFCAirPmCtrl_read/1 -> Terminator_35/1
  VFCAutoDefrstPopUp_VFCAutoDefrstPopUp_read/1 -> Terminator_36/1
  VFCAutoDeHumPopup_VFCAutoDeHumPopup_read/1 -> Terminator_37/1
  VFCInfoPushInCarCDSMon_VFCInfoPushInCarCDSMon_read/1 -> Terminator_38/1
  CarTiGlb_Ngcc_CarTiGlb_read/1 -> Terminator_39/1
  CarTiGlb_Ngcc_CarTiGlb_IsUpdated_read/1 -> Terminator_40/1
  ClimaAtRowSecActvNgcc_ClimaAtRowSecActv_read/1 -> Terminator_41/1
  ClimaAtRowSecActvNgcc_ClimaAtRowSecActvLe_read/1 -> Terminator_42/1
  ClimaAtRowSecActvNgcc_ClimaAtRowSecActvRi_read/1 -> Terminator_43/1
  ClimaCfgForErev1_ClimaCfgForErev1_read/1 -> Terminator_44/1
  UInt16forCompile_UInt16forCompile_read/1 -> Terminator_45/1
  Ground_1/1 -> ActvnTiForClimaCtrlRe_ActvnTiForClimaCtrlRe_write/1
  Ground_2/1 -> TiOfDeactvnForClimaCtrlRe_TiOfDeactvnForClimaCtrlRe_write/1
  Ground_3/1 -> TiOfDeactvnForCmptmtFanRe_TiOfDeactvnForCmptmtFanRe_write/1
  Ground_4/1 -> ActvnTiForClimaCtrl_ActvnTiForClimaCtrl_write/1
  Ground_5/1 -> CarTiIntForClima_CarTiIntForClima_write/1
  Ground_6/1 -> ClimaActvnInhb_ClimaActvnInhb_write/1
  Ground_7/1 -> ClimaTiMgrVFCInfotainmentPush_ClimaTiMgrVFCInfotainmentPush_write/1
  Ground_8/1 -> HmiPassTempSyncSetFlag_HmiPassTempSyncSetFlag_write/1
  Ground_9/1 -> MemSaveFlgForClimaTiMgr_MemSaveFlgForClimaTiMgr_write/1
  Ground_10/1(Out1) -> MemWrForClimaTiMgr_MemWrForClimaTiMgr_write/1
  Ground_11/1 -> SysDeepSlpTi_SysDeepSlpTi_write/1
  Ground_12/1 -> TiOfDeactvnForClimaCtrlNgcc_TiOfDeactvnForClimaCtrl_write/1
  Ground_13/1 -> TiOfDeactvnForClimaObs_TiOfDeactvnForClimaObs_write/1
  Ground_14/1 -> TiOfDeactvnForCmptmtFan_TiOfDeactvnForCmptmtFan_write/1
  Ground_15/1 -> WinRfClsdReqForCoolgDwnInt_WinRfClsdReqForCoolgDwnInt_write/1

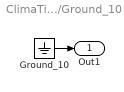
== system ClimaTiMgr/ClimaTiMgr_Runnable_sys/Ground_10 ==
  Ground_10 : Ground
  Out1 : Outport  OutDataTypeStr=Bus: MemRecForClimaTiMgr  PortDimensions=1
  wires:
  Ground_10/1 -> Out1/1
